simulink model "MX_Gateway"  (Simulink 9.0)
settings: Solver=FixedStepDiscrete  StartTime=0.0  StopTime=100  FixedStep=0.01  RelTol=1e-3
values from neighbouring files (symbols this model uses):
  ACTIVE = Bobcat.Parameter; CreateDefine( ACTIVE                         ,1                          ,'Define' ,'defines.h' )    [defines.m]
  ACTIVE_MODE_ONE = Bobcat.Parameter; CreateDefine( ACTIVE_MODE_ONE                ,19                         ,'Define' ,'defines.h' )    [defines.m]
  AUTHENTICATED = Bobcat.Parameter; CreateDefine( AUTHENTICATED                  ,1                          ,'Define' ,'defines.h' )    [defines.m]
  AUX_EMEA_CONTROLS = Bobcat.Parameter; CreateDefine( AUX_EMEA_CONTROLS  ,1   ,'Define' ,'defines.h' )    [enums.m]
  AUX_FLOW_HIGH = Bobcat.Parameter; CreateDefine( AUX_FLOW_HIGH           ,4  ,'Define' ,'defines.h' )    [enums.m]
  AUX_FLOW_INACTIVE = Bobcat.Parameter; CreateDefine( AUX_FLOW_INACTIVE       ,0  ,'Define' ,'defines.h' )    [enums.m]
  AUX_FLOW_LOW = Bobcat.Parameter; CreateDefine( AUX_FLOW_LOW            ,1  ,'Define' ,'defines.h' )    [enums.m]
  AUX_FLOW_MEDIUM = Bobcat.Parameter; CreateDefine( AUX_FLOW_MEDIUM         ,2  ,'Define' ,'defines.h' )    [enums.m]
  AUX_FLOW_STANDARD = Bobcat.Parameter; CreateDefine( AUX_FLOW_STANDARD       ,3  ,'Define' ,'defines.h' )    [enums.m]
  AUX_NON_FUNCTIONAL = Bobcat.Parameter; CreateDefine( AUX_NON_FUNCTIONAL ,255 ,'Define' ,'defines.h' )    [enums.m]
  BASE_ACTIVE = Bobcat.Parameter; CreateDefine( BASE_ACTIVE                    ,253                        ,'Define' ,'defines.h' )    [defines.m]
  BASE_DETENT = Bobcat.Parameter; CreateDefine( BASE_DETENT                    ,1                          ,'Define' ,'defines.h' )    [defines.m]
  BEEP_NONE = Bobcat.Parameter; CreateDefine( BEEP_NONE                      ,255                        ,'Define' ,'defines.h' )    [defines.m]
  CD_AIR_FILTER_DEBOUNCE_NORMAL = Bobcat.Parameter; CreateAliasCD( CD_AIR_FILTER_DEBOUNCE_NORMAL                        ,uint16(500)                ,'ConstantDataPointer' ,'CONSTANT_DATA' ,'AIR_FILTER_DEBOUNCE_NORMAL')    [cdata.m]    (also in hc12_cdata.m; m4_cdata.m)
  CD_AIR_FILTER_DEBOUNCE_NOT_CONNECTED = Bobcat.Parameter; CreateAliasCD( CD_AIR_FILTER_DEBOUNCE_NOT_CONNECTED                 ,uint16(3000)               ,'ConstantDataPointer' ,'CONSTANT_DATA' ,'AIR_FILTER_DEBOUNCE_NOT_CONNECTED')    [cdata.m]    (also in hc12_cdata.m; m4_cdata.m)
  CD_AIR_FILTER_DEBOUNCE_PLUGGED = Bobcat.Parameter; CreateAliasCD( CD_AIR_FILTER_DEBOUNCE_PLUGGED                       ,uint16(3000)               ,'ConstantDataPointer' ,'CONSTANT_DATA' ,'AIR_FILTER_DEBOUNCE_PLUGGED')    [cdata.m]    (also in hc12_cdata.m; m4_cdata.m)
  CD_AIR_FILTER_FUNCTION = Bobcat.Parameter; CreateAliasCD( CD_AIR_FILTER_FUNCTION                               ,uint8(255)                 ,'ConstantDataPointer' ,'CONSTANT_DATA' ,'AIR_FILTER_FUNCTION')    [cdata.m]    (also in hc12_cdata.m; m4_cdata.m)
  CD_AIR_FILTER_PLUGGED_WEIGHT = Bobcat.Parameter; CreateAliasCD( CD_AIR_FILTER_PLUGGED_WEIGHT                         ,uint8(2)                   ,'ConstantDataPointer' ,'CONSTANT_DATA' ,'AIR_FILTER_PLUGGED_WEIGHT')    [cdata.m]    (also in hc12_cdata.m; m4_cdata.m)
  CD_AIR_FILTER_UNPLUGGED_WEIGHT = Bobcat.Parameter; CreateAliasCD( CD_AIR_FILTER_UNPLUGGED_WEIGHT                       ,uint8(1)                   ,'ConstantDataPointer' ,'CONSTANT_DATA' ,'AIR_FILTER_UNPLUGGED_WEIGHT')    [cdata.m]    (also in hc12_cdata.m; m4_cdata.m)
  CD_AUTOIDLE_ARMBAR_DELAY = Bobcat.Parameter; CreateAliasCD( CD_AUTOIDLE_ARMBAR_DELAY                             ,uint8(2)                   ,'ConstantDataPointer' ,'CONSTANT_DATA' ,'AUTOIDLE_ARMBAR_DELAY')    [cdata.m]    (also in hc12_cdata.m; m4_cdata.m)
  CD_AUTOIDLE_FUNCTION = Bobcat.Parameter; CreateAliasCD( CD_AUTOIDLE_FUNCTION                                 ,uint8(255)                 ,'ConstantDataPointer' ,'CONSTANT_DATA' ,'AUTOIDLE_FUNCTION')    [cdata.m]    (also in hc12_cdata.m; m4_cdata.m)
  CD_AUTOIDLE_IDLE_DELAY = Bobcat.Parameter; CreateAliasCD( CD_AUTOIDLE_IDLE_DELAY                               ,uint8(40)                  ,'ConstantDataPointer' ,'CONSTANT_DATA' ,'AUTOIDLE_IDLE_DELAY')    [cdata.m]    (also in hc12_cdata.m; m4_cdata.m)
  CD_AUTOIDLE_LOW_IDLE_START = Bobcat.Parameter; CreateAliasCD( CD_AUTOIDLE_LOW_IDLE_START                           ,uint8(255)                 ,'ConstantDataPointer' ,'CONSTANT_DATA' ,'AUTOIDLE_LOW_IDLE_START')    [cdata.m]    (also in hc12_cdata.m; m4_cdata.m)
  CD_AUTOIDLE_PRESSURE_OFF = Bobcat.Parameter; CreateAliasCD( CD_AUTOIDLE_PRESSURE_OFF                             ,uint16(300)                ,'ConstantDataPointer' ,'CONSTANT_DATA' ,'AUTOIDLE_PRESSURE_OFF')    [cdata.m]    (also in hc12_cdata.m; m4_cdata.m)
  CD_AUTOIDLE_PRESSURE_ON = Bobcat.Parameter; CreateAliasCD( CD_AUTOIDLE_PRESSURE_ON                              ,uint16(270)                ,'ConstantDataPointer' ,'CONSTANT_DATA' ,'AUTOIDLE_PRESSURE_ON')    [cdata.m]    (also in hc12_cdata.m; m4_cdata.m)
  CD_AUTOSHIFT_FUNCTION = Bobcat.Parameter; CreateAliasCD( CD_AUTOSHIFT_FUNCTION                                ,uint8(255)                 ,'ConstantDataPointer' ,'CONSTANT_DATA' ,'AUTOSHIFT_FUNCTION')    [cdata.m]    (also in hc12_cdata.m; m4_cdata.m)
  CD_AUTOSHIFT_PHIGH1 = Bobcat.Parameter; CreateAliasCD( CD_AUTOSHIFT_PHIGH1                                  ,uint16(205)                ,'ConstantDataPointer' ,'CONSTANT_DATA' ,'AUTOSHIFT_PHIGH1')    [cdata.m]    (also in hc12_cdata.m; m4_cdata.m)
  CD_AUTOSHIFT_PHIGH2 = Bobcat.Parameter; CreateAliasCD( CD_AUTOSHIFT_PHIGH2                                  ,uint16(193)                ,'ConstantDataPointer' ,'CONSTANT_DATA' ,'AUTOSHIFT_PHIGH2')    [cdata.m]    (also in hc12_cdata.m; m4_cdata.m)
  CD_AUTOSHIFT_PHIGH_TEMP1 = Bobcat.Parameter; CreateAliasCD( CD_AUTOSHIFT_PHIGH_TEMP1                             ,int16(70)                  ,'ConstantDataPointer' ,'CONSTANT_DATA' ,'AUTOSHIFT_PHIGH_TEMP1')    [cdata.m]    (also in hc12_cdata.m; m4_cdata.m)
  CD_AUTOSHIFT_PHIGH_TEMP2 = Bobcat.Parameter; CreateAliasCD( CD_AUTOSHIFT_PHIGH_TEMP2                             ,int16(160)                 ,'ConstantDataPointer' ,'CONSTANT_DATA' ,'AUTOSHIFT_PHIGH_TEMP2')    [cdata.m]    (also in hc12_cdata.m; m4_cdata.m)
  CD_AUTOSHIFT_PLOW1 = Bobcat.Parameter; CreateAliasCD( CD_AUTOSHIFT_PLOW1                                   ,uint16(130)                ,'ConstantDataPointer' ,'CONSTANT_DATA' ,'AUTOSHIFT_PLOW1')    [cdata.m]    (also in hc12_cdata.m; m4_cdata.m)
  CD_AUTOSHIFT_PLOW2 = Bobcat.Parameter; CreateAliasCD( CD_AUTOSHIFT_PLOW2                                   ,uint16(100)                ,'ConstantDataPointer' ,'CONSTANT_DATA' ,'AUTOSHIFT_PLOW2')    [cdata.m]    (also in hc12_cdata.m; m4_cdata.m)
  CD_AUTOSHIFT_PLOW_TEMP1 = Bobcat.Parameter; CreateAliasCD( CD_AUTOSHIFT_PLOW_TEMP1                              ,int16(20)                  ,'ConstantDataPointer' ,'CONSTANT_DATA' ,'AUTOSHIFT_PLOW_TEMP1')    [cdata.m]    (also in hc12_cdata.m; m4_cdata.m)
  CD_AUTOSHIFT_PLOW_TEMP2 = Bobcat.Parameter; CreateAliasCD( CD_AUTOSHIFT_PLOW_TEMP2                              ,int16(80)                  ,'ConstantDataPointer' ,'CONSTANT_DATA' ,'AUTOSHIFT_PLOW_TEMP2')    [cdata.m]    (also in hc12_cdata.m; m4_cdata.m)
  CD_AUTOSHIFT_TRAVEL_DELAY = Bobcat.Parameter; CreateAliasCD( CD_AUTOSHIFT_TRAVEL_DELAY                            ,uint8(32)                  ,'ConstantDataPointer' ,'CONSTANT_DATA' ,'AUTOSHIFT_TRAVEL_DELAY')    [cdata.m]    (also in hc12_cdata.m; m4_cdata.m)
  CD_AUX2_CRACKPOINT_CURRENT = Bobcat.Parameter; CreateAliasCD( CD_AUX2_CRACKPOINT_CURRENT                           ,fi(0,0,16,12,'hex','0c28') ,'ConstantDataPointer' ,'CONSTANT_DATA' ,'AUX2_CRACKPOINT_CURRENT')    [cdata.m]    (also in hc12_cdata.m; m4_cdata.m)
  CD_AUX2_FLOW_LOW_FUNCTION = Bobcat.Parameter; CreateAliasCD( CD_AUX2_FLOW_LOW_FUNCTION                            ,uint8(255)                 ,'ConstantDataPointer' ,'CONSTANT_DATA' ,'AUX2_FLOW_LOW_FUNCTION')    [cdata.m]    (also in hc12_cdata.m; m4_cdata.m)
  CD_AUX2_FLOW_MED_FUNCTION = Bobcat.Parameter; CreateAliasCD( CD_AUX2_FLOW_MED_FUNCTION                            ,uint8(255)                 ,'ConstantDataPointer' ,'CONSTANT_DATA' ,'AUX2_FLOW_MED_FUNCTION')    [cdata.m]    (also in hc12_cdata.m; m4_cdata.m)
  CD_AUX2_HI_FLOW_PERCENT = Bobcat.Parameter; CreateAliasCD( CD_AUX2_HI_FLOW_PERCENT                              ,fi(0,0,16,16,'hex','ffff') ,'ConstantDataPointer' ,'CONSTANT_DATA' ,'AUX2_HI_FLOW_PERCENT')    [cdata.m]    (also in hc12_cdata.m; m4_cdata.m)
  CD_AUX2_LOW_FLOW_PERCENT = Bobcat.Parameter; CreateAliasCD( CD_AUX2_LOW_FLOW_PERCENT                             ,fi(0,0,16,16,'hex','7333') ,'ConstantDataPointer' ,'CONSTANT_DATA' ,'AUX2_LOW_FLOW_PERCENT')    [cdata.m]    (also in hc12_cdata.m; m4_cdata.m)
  CD_AUX2_MAX_FLOW_CURRENT = Bobcat.Parameter; CreateAliasCD( CD_AUX2_MAX_FLOW_CURRENT                             ,fi(0,0,16,12,'hex','14cc') ,'ConstantDataPointer' ,'CONSTANT_DATA' ,'AUX2_MAX_FLOW_CURRENT')    [cdata.m]    (also in hc12_cdata.m; m4_cdata.m)
  CD_AUX2_MED_FLOW_PERCENT = Bobcat.Parameter; CreateAliasCD( CD_AUX2_MED_FLOW_PERCENT                             ,fi(0,0,16,16,'hex','9999') ,'ConstantDataPointer' ,'CONSTANT_DATA' ,'AUX2_MED_FLOW_PERCENT')    [cdata.m]    (also in hc12_cdata.m; m4_cdata.m)
  CD_AUX_CRACKPOINT_CURRENT = Bobcat.Parameter; CreateAliasCD( CD_AUX_CRACKPOINT_CURRENT                            ,fi(0,0,16,12,'hex','0000') ,'ConstantDataPointer' ,'CONSTANT_DATA' ,'AUX_CRACKPOINT_CURRENT')    [cdata.m]    (also in hc12_cdata.m; m4_cdata.m)
  CD_AUX_DETENT_FLASH_TIME = Bobcat.Parameter; CreateAliasCD( CD_AUX_DETENT_FLASH_TIME                             ,uint16(72)                 ,'ConstantDataPointer' ,'CONSTANT_DATA' ,'AUX_DETENT_FLASH_TIME')    [cdata.m]    (also in hc12_cdata.m; m4_cdata.m)
  CD_AUX_DETENT_HOLD_TIME = Bobcat.Parameter; CreateAliasCD( CD_AUX_DETENT_HOLD_TIME                              ,uint16(100)                ,'ConstantDataPointer' ,'CONSTANT_DATA' ,'AUX_DETENT_HOLD_TIME')    [cdata.m]    (also in hc12_cdata.m; m4_cdata.m)
  CD_AUX_FULL_ON_AUX1 = Bobcat.Parameter; CreateAliasCD( CD_AUX_FULL_ON_AUX1                                  ,fi(0,0,16,12,'hex','10cd') ,'ConstantDataPointer' ,'CONSTANT_DATA' ,'AUX_FULL_ON_AUX1')    [cdata.m]    (also in hc12_cdata.m; m4_cdata.m)
  CD_AUX_FULL_ON_AUX2 = Bobcat.Parameter; CreateAliasCD( CD_AUX_FULL_ON_AUX2                                  ,fi(0,0,16,12,'hex','139d') ,'ConstantDataPointer' ,'CONSTANT_DATA' ,'AUX_FULL_ON_AUX2')    [cdata.m]    (also in hc12_cdata.m; m4_cdata.m)
  CD_AUX_FULL_ON_AUX3 = Bobcat.Parameter; CreateAliasCD( CD_AUX_FULL_ON_AUX3                                  ,fi(0,0,16,12,'hex','17d7') ,'ConstantDataPointer' ,'CONSTANT_DATA' ,'AUX_FULL_ON_AUX3')    [cdata.m]    (also in hc12_cdata.m; m4_cdata.m)
  CD_AUX_FUNCTION = Bobcat.Parameter; CreateAliasCD( CD_AUX_FUNCTION                                      ,uint8(0)                   ,'ConstantDataPointer' ,'CONSTANT_DATA' ,'AUX_FUNCTION')    [cdata.m]    (also in hc12_cdata.m; m4_cdata.m)
  CD_AUX_RATE_LIMIT_CRACKPOINT = Bobcat.Parameter; CreateAliasCD( CD_AUX_RATE_LIMIT_CRACKPOINT                         ,fi(0,0,16,12,'hex','0800') ,'ConstantDataPointer' ,'CONSTANT_DATA' ,'AUX_RATE_LIMIT_CRACKPOINT')    [cdata.m]    (also in hc12_cdata.m; m4_cdata.m)
  CD_AUX_RATE_LIMIT_CRACKPOINT_RATE = Bobcat.Parameter; CreateAliasCD( CD_AUX_RATE_LIMIT_CRACKPOINT_RATE                    ,fi(0,1,32,12,'int',102400) ,'ConstantDataPointer' ,'CONSTANT_DATA' ,'AUX_RATE_LIMIT_CRACKPOINT_RATE')    [cdata.m]    (also in hc12_cdata.m; m4_cdata.m)
  CD_AUX_RATE_LIMIT_FUNCTION = Bobcat.Parameter; CreateAliasCD( CD_AUX_RATE_LIMIT_FUNCTION                           ,uint8(255)                 ,'ConstantDataPointer' ,'CONSTANT_DATA' ,'AUX_RATE_LIMIT_FUNCTION')    [cdata.m]    (also in hc12_cdata.m; m4_cdata.m)
  CD_AUX_RATE_LIMIT_MAX_ENG_SPD = Bobcat.Parameter; CreateAliasCD( CD_AUX_RATE_LIMIT_MAX_ENG_SPD                        ,uint16(1600)               ,'ConstantDataPointer' ,'CONSTANT_DATA' ,'AUX_RATE_LIMIT_MAX_ENG_SPD')    [cdata.m]    (also in hc12_cdata.m; m4_cdata.m)
  CD_AUX_RATE_LIMIT_MIN_ENG_SPD = Bobcat.Parameter; CreateAliasCD( CD_AUX_RATE_LIMIT_MIN_ENG_SPD                        ,uint16(100)                ,'ConstantDataPointer' ,'CONSTANT_DATA' ,'AUX_RATE_LIMIT_MIN_ENG_SPD')    [cdata.m]    (also in hc12_cdata.m; m4_cdata.m)
  CD_AUX_RATE_LIMIT_NORMAL_RATE = Bobcat.Parameter; CreateAliasCD( CD_AUX_RATE_LIMIT_NORMAL_RATE                        ,fi(0,1,32,12,'int',2048)   ,'ConstantDataPointer' ,'CONSTANT_DATA' ,'AUX_RATE_LIMIT_NORMAL_RATE')    [cdata.m]    (also in hc12_cdata.m; m4_cdata.m)
  CD_AUXPOT_CAL_DEADBAND_HIGH = Bobcat.Parameter; CreateAliasCD( CD_AUXPOT_CAL_DEADBAND_HIGH                          ,uint16(574)                ,'ConstantDataPointer' ,'CONSTANT_DATA' ,'AUXPOT_CAL_DEADBAND_HIGH')    [cdata.m]    (also in hc12_cdata.m; m4_cdata.m)
  CD_AUXPOT_CAL_DEADBAND_LOW = Bobcat.Parameter; CreateAliasCD( CD_AUXPOT_CAL_DEADBAND_LOW                           ,uint16(424)                ,'ConstantDataPointer' ,'CONSTANT_DATA' ,'AUXPOT_CAL_DEADBAND_LOW')    [cdata.m]    (also in hc12_cdata.m; m4_cdata.m)
  CD_AUXPOT_CENTER_RATIO = Bobcat.Parameter; CreateAliasCD( CD_AUXPOT_CENTER_RATIO                               ,uint16(500)                ,'ConstantDataPointer' ,'CONSTANT_DATA' ,'AUXPOT_CENTER_RATIO')    [cdata.m]    (also in hc12_cdata.m; m4_cdata.m)
  CD_AUXPOT_DEADBAND = Bobcat.Parameter; CreateAliasCD( CD_AUXPOT_DEADBAND                                   ,uint8(38)                  ,'ConstantDataPointer' ,'CONSTANT_DATA' ,'AUXPOT_DEADBAND')    [cdata.m]    (also in hc12_cdata.m; m4_cdata.m)
  CD_AUXPOT_FUNCTION = Bobcat.Parameter; CreateAliasCD( CD_AUXPOT_FUNCTION                                   ,uint8(0)                   ,'ConstantDataPointer' ,'CONSTANT_DATA' ,'AUXPOT_FUNCTION')    [cdata.m]    (also in hc12_cdata.m; m4_cdata.m)
  CD_AUXPOT_RATIO_GAIN = Bobcat.Parameter; CreateAliasCD( CD_AUXPOT_RATIO_GAIN                                 ,uint16(1000)               ,'ConstantDataPointer' ,'CONSTANT_DATA' ,'AUXPOT_RATIO_GAIN')    [cdata.m]    (also in hc12_cdata.m; m4_cdata.m)
  CD_CAN_THROTTLE_FUNCTION = Bobcat.Parameter; CreateAliasCD( CD_CAN_THROTTLE_FUNCTION                             ,uint8(255)                 ,'ConstantDataPointer' ,'CONSTANT_DATA' ,'CAN_THROTTLE_FUNCTION')    [cdata.m]    (also in hc12_cdata.m; m4_cdata.m)
  CD_CAN_THROTTLE_REVERSE = Bobcat.Parameter; CreateAliasCD( CD_CAN_THROTTLE_REVERSE                              ,uint8(255)                 ,'ConstantDataPointer' ,'CONSTANT_DATA' ,'CAN_THROTTLE_REVERSE')    [cdata.m]    (also in hc12_cdata.m; m4_cdata.m)
  CD_CONSOLE_DEBOUNCE_ABSENT = Bobcat.Parameter; CreateAliasCD( CD_CONSOLE_DEBOUNCE_ABSENT                           ,uint16(12)                 ,'ConstantDataPointer' ,'CONSTANT_DATA' ,'CONSOLE_DEBOUNCE_ABSENT')    [cdata.m]    (also in hc12_cdata.m; m4_cdata.m)
  CD_CONSOLE_DEBOUNCE_OORH = Bobcat.Parameter; CreateAliasCD( CD_CONSOLE_DEBOUNCE_OORH                             ,uint16(12)                 ,'ConstantDataPointer' ,'CONSTANT_DATA' ,'CONSOLE_DEBOUNCE_OORH')    [cdata.m]    (also in hc12_cdata.m; m4_cdata.m)
  CD_CONSOLE_DEBOUNCE_OORL = Bobcat.Parameter; CreateAliasCD( CD_CONSOLE_DEBOUNCE_OORL                             ,uint16(12)                 ,'ConstantDataPointer' ,'CONSTANT_DATA' ,'CONSOLE_DEBOUNCE_OORL')    [cdata.m]    (also in hc12_cdata.m; m4_cdata.m)
  CD_CONSOLE_DEBOUNCE_PRESENT = Bobcat.Parameter; CreateAliasCD( CD_CONSOLE_DEBOUNCE_PRESENT                          ,uint16(12)                 ,'ConstantDataPointer' ,'CONSTANT_DATA' ,'CONSOLE_DEBOUNCE_PRESENT')    [cdata.m]    (also in hc12_cdata.m; m4_cdata.m)
  CD_CONSOLE_FUNCTION = Bobcat.Parameter; CreateAliasCD( CD_CONSOLE_FUNCTION                                  ,uint8(0)                   ,'ConstantDataPointer' ,'CONSTANT_DATA' ,'CONSOLE_FUNCTION')    [cdata.m]    (also in hc12_cdata.m; m4_cdata.m)
  CD_CONSOLE_OORH = Bobcat.Parameter; CreateAliasCD( CD_CONSOLE_OORH                                      ,uint16(740)                ,'ConstantDataPointer' ,'CONSTANT_DATA' ,'CONSOLE_OORH')    [cdata.m]    (also in hc12_cdata.m; m4_cdata.m)
  CD_CONSOLE_OORL = Bobcat.Parameter; CreateAliasCD( CD_CONSOLE_OORL                                      ,uint16(10)                 ,'ConstantDataPointer' ,'CONSTANT_DATA' ,'CONSOLE_OORL')    [cdata.m]    (also in hc12_cdata.m; m4_cdata.m)
  CD_CONSOLE_PRESENT_HIGH = Bobcat.Parameter; CreateAliasCD( CD_CONSOLE_PRESENT_HIGH                              ,uint16(515)                ,'ConstantDataPointer' ,'CONSTANT_DATA' ,'CONSOLE_PRESENT_HIGH')    [cdata.m]    (also in hc12_cdata.m; m4_cdata.m)
  CD_CONSOLE_PRESENT_LOW = Bobcat.Parameter; CreateAliasCD( CD_CONSOLE_PRESENT_LOW                               ,uint16(375)                ,'ConstantDataPointer' ,'CONSTANT_DATA' ,'CONSOLE_PRESENT_LOW')    [cdata.m]    (also in hc12_cdata.m; m4_cdata.m)
  CD_DELUXE_GEN5_5_FUNCTION = Bobcat.Parameter; CreateAliasCD( CD_DELUXE_GEN5_5_FUNCTION                            ,uint8(255)                 ,'ConstantDataPointer' ,'CONSTANT_DATA' ,'DELUXE_GEN5_5_FUNCTION')    [cdata.m]    (also in hc12_cdata.m; m4_cdata.m)
  CD_DELUXE_GEN5_FUNCTION = Bobcat.Parameter; CreateAliasCD( CD_DELUXE_GEN5_FUNCTION                              ,uint8(255)                 ,'ConstantDataPointer' ,'CONSTANT_DATA' ,'DELUXE_GEN5_FUNCTION')    [cdata.m]    (also in hc12_cdata.m; m4_cdata.m)
  CD_DERATE_ENGINE_SPEED_DEBOUNCE_HIGH = Bobcat.Parameter; CreateAliasCD( CD_DERATE_ENGINE_SPEED_DEBOUNCE_HIGH                 ,uint16(200)                ,'ConstantDataPointer' ,'CONSTANT_DATA' ,'DERATE_ENGINE_SPEED_DEBOUNCE_HIGH')    [cdata.m]    (also in hc12_cdata.m; m4_cdata.m)
  CD_DERATE_ENGINE_SPEED_DEBOUNCE_SHUTDOWN = Bobcat.Parameter; CreateAliasCD( CD_DERATE_ENGINE_SPEED_DEBOUNCE_SHUTDOWN             ,uint16(1000)               ,'ConstantDataPointer' ,'CONSTANT_DATA' ,'DERATE_ENGINE_SPEED_DEBOUNCE_SHUTDOWN')    [cdata.m]    (also in hc12_cdata.m; m4_cdata.m)
  CD_DERATE_ENGINE_SPEED_HIGH_OFFSET = Bobcat.Parameter; CreateAliasCD( CD_DERATE_ENGINE_SPEED_HIGH_OFFSET                   ,uint16(200)                ,'ConstantDataPointer' ,'CONSTANT_DATA' ,'DERATE_ENGINE_SPEED_HIGH_OFFSET')    [cdata.m]    (also in hc12_cdata.m; m4_cdata.m)
  CD_DERATE_METHOD = Bobcat.Parameter; CreateAliasCD( CD_DERATE_METHOD                                     ,uint8(0)                   ,'ConstantDataPointer' ,'CONSTANT_DATA' ,'DERATE_METHOD')    [cdata.m]    (also in hc12_cdata.m; m4_cdata.m)
  CD_DUMP_VALVE_FUNCTION = Bobcat.Parameter; CreateAliasCD( CD_DUMP_VALVE_FUNCTION                               ,uint8(255)                 ,'ConstantDataPointer' ,'CONSTANT_DATA' ,'DUMP_VALVE_FUNCTION')    [cdata.m]    (also in hc12_cdata.m; m4_cdata.m)
  CD_DUMP_VALVE_ON_CURRENT = Bobcat.Parameter; CreateAliasCD( CD_DUMP_VALVE_ON_CURRENT                             ,fi(0,0,16,12,'hex','ffff') ,'ConstantDataPointer' ,'CONSTANT_DATA' ,'DUMP_VALVE_ON_CURRENT')    [cdata.m]    (also in hc12_cdata.m; m4_cdata.m)
  CD_ECO_MODE_THROTTLE_REDUCTION = Bobcat.Parameter; CreateAliasCD( CD_ECO_MODE_THROTTLE_REDUCTION                       ,uint16(850)                ,'ConstantDataPointer' ,'CONSTANT_DATA' ,'ECO_MODE_THROTTLE_REDUCTION')    [cdata.m]    (also in hc12_cdata.m; m4_cdata.m)
  CD_ECT_FILTER_GAIN = Bobcat.Parameter; CreateAliasCD( CD_ECT_FILTER_GAIN                                   ,uint8(6)                   ,'ConstantDataPointer' ,'CONSTANT_DATA' ,'ECT_FILTER_GAIN')    [cdata.m]    (also in hc12_cdata.m; m4_cdata.m)
  CD_ECU_FUNCTION = Bobcat.Parameter; CreateAliasCD( CD_ECU_FUNCTION                                      ,uint8(255)                 ,'ConstantDataPointer' ,'CONSTANT_DATA' ,'ECU_FUNCTION')    [cdata.m]    (also in hc12_cdata.m; m4_cdata.m)
  CD_ECU_HOLD_DEBOUNCE_ERROR_ON = Bobcat.Parameter; CreateAliasCD( CD_ECU_HOLD_DEBOUNCE_ERROR_ON                        ,uint16(20)                 ,'ConstantDataPointer' ,'CONSTANT_DATA' ,'ECU_HOLD_DEBOUNCE_ERROR_ON')    [cdata.m]    (also in hc12_cdata.m; m4_cdata.m)
  CD_EMEA_CONTROLS_HOLD_TIME = Bobcat.Parameter; CreateAliasCD( CD_EMEA_CONTROLS_HOLD_TIME                           ,uint16(30)                 ,'ConstantDataPointer' ,'CONSTANT_DATA' ,'EMEA_CONTROLS_HOLD_TIME')    [cdata.m]    (also in hc12_cdata.m; m4_cdata.m)
  CD_EMEA_CONTROLS_OPTION_A = Bobcat.Parameter; CreateAliasCD( CD_EMEA_CONTROLS_OPTION_A                            ,uint8(255)                 ,'ConstantDataPointer' ,'CONSTANT_DATA' ,'EMEA_CONTROLS_OPTION_A')    [cdata.m]    (also in hc12_cdata.m; m4_cdata.m)
  CD_EMEA_CONTROLS_OPTION_C = Bobcat.Parameter; CreateAliasCD( CD_EMEA_CONTROLS_OPTION_C                            ,uint8(255)                 ,'ConstantDataPointer' ,'CONSTANT_DATA' ,'EMEA_CONTROLS_OPTION_C')    [cdata.m]    (also in hc12_cdata.m; m4_cdata.m)
  CD_EMEA_CONTROLS_REVERSE = Bobcat.Parameter; CreateAliasCD( CD_EMEA_CONTROLS_REVERSE                             ,uint8(255)                 ,'ConstantDataPointer' ,'CONSTANT_DATA' ,'EMEA_CONTROLS_REVERSE')    [cdata.m]    (also in hc12_cdata.m; m4_cdata.m)
  CD_EMEA_ELECTRIC_VERSION = Bobcat.Parameter; CreateAliasCD( CD_EMEA_ELECTRIC_VERSION                             ,uint8(0)                   ,'ConstantDataPointer' ,'CONSTANT_DATA' ,'EMEA_ELECTRIC_VERSION')    [cdata.m]    (also in hc12_cdata.m; m4_cdata.m)
  CD_EMEA_POT_CAL_DEADBAND_HIGH = Bobcat.Parameter; CreateAliasCD( CD_EMEA_POT_CAL_DEADBAND_HIGH                        ,uint16(574)                ,'ConstantDataPointer' ,'CONSTANT_DATA' ,'EMEA_POT_CAL_DEADBAND_HIGH')    [cdata.m]    (also in hc12_cdata.m; m4_cdata.m)
  CD_EMEA_POT_CAL_DEADBAND_LOW = Bobcat.Parameter; CreateAliasCD( CD_EMEA_POT_CAL_DEADBAND_LOW                         ,uint16(424)                ,'ConstantDataPointer' ,'CONSTANT_DATA' ,'EMEA_POT_CAL_DEADBAND_LOW')    [cdata.m]    (also in hc12_cdata.m; m4_cdata.m)
  CD_ENGINE_RPM_FACTOR = Bobcat.Parameter; CreateAliasCD( CD_ENGINE_RPM_FACTOR                                 ,uint8(35)                  ,'ConstantDataPointer' ,'CONSTANT_DATA' ,'ENGINE_RPM_FACTOR')    [cdata.m]    (also in hc12_cdata.m; m4_cdata.m)
  CD_ENGINE_SPEED_FILTER_FAST_VALUE = Bobcat.Parameter; CreateAliasCD( CD_ENGINE_SPEED_FILTER_FAST_VALUE                    ,uint8(1)                   ,'ConstantDataPointer' ,'CONSTANT_DATA' ,'ENGINE_SPEED_FILTER_FAST_VALUE')    [cdata.m]    (also in hc12_cdata.m; m4_cdata.m)
  CD_ENGINE_SPEED_FILTER_SLOW_VALUE = Bobcat.Parameter; CreateAliasCD( CD_ENGINE_SPEED_FILTER_SLOW_VALUE                    ,uint8(4)                   ,'ConstantDataPointer' ,'CONSTANT_DATA' ,'ENGINE_SPEED_FILTER_SLOW_VALUE')    [cdata.m]    (also in hc12_cdata.m; m4_cdata.m)
  CD_ENGINE_SPEED_RUNNING = Bobcat.Parameter; CreateAliasCD( CD_ENGINE_SPEED_RUNNING                              ,uint16(800)                ,'ConstantDataPointer' ,'CONSTANT_DATA' ,'ENGINE_SPEED_RUNNING')    [cdata.m]    (also in hc12_cdata.m; m4_cdata.m)
  CD_ENGINE_START_DELAY_DEBOUNCE_RPM_NO_SIGNAL = Bobcat.Parameter; CreateAliasCD( CD_ENGINE_START_DELAY_DEBOUNCE_RPM_NO_SIGNAL         ,uint16(0)                  ,'ConstantDataPointer' ,'CONSTANT_DATA' ,'ENGINE_START_DELAY_DEBOUNCE_RPM_NO_SIGNAL')    [cdata.m]    (also in hc12_cdata.m; m4_cdata.m)
  CD_ENGINE_START_DELAY_OFF_TIME = Bobcat.Parameter; CreateAliasCD( CD_ENGINE_START_DELAY_OFF_TIME                       ,uint16(0)                  ,'ConstantDataPointer' ,'CONSTANT_DATA' ,'ENGINE_START_DELAY_OFF_TIME')    [cdata.m]    (also in hc12_cdata.m; m4_cdata.m)
  CD_ENGINE_START_DELAY_RPM_NO_SIGNAL = Bobcat.Parameter; CreateAliasCD( CD_ENGINE_START_DELAY_RPM_NO_SIGNAL                  ,uint16(0)                  ,'ConstantDataPointer' ,'CONSTANT_DATA' ,'ENGINE_START_DELAY_RPM_NO_SIGNAL')    [cdata.m]    (also in hc12_cdata.m; m4_cdata.m)
  CD_EOP_DEBOUNCE_EXTREMELY_LOW = Bobcat.Parameter; CreateAliasCD( CD_EOP_DEBOUNCE_EXTREMELY_LOW                        ,uint16(50)                 ,'ConstantDataPointer' ,'CONSTANT_DATA' ,'EOP_DEBOUNCE_EXTREMELY_LOW')    [cdata.m]    (also in hc12_cdata.m; m4_cdata.m)
  CD_EOP_DEBOUNCE_NORMAL = Bobcat.Parameter; CreateAliasCD( CD_EOP_DEBOUNCE_NORMAL                               ,uint16(50)                 ,'ConstantDataPointer' ,'CONSTANT_DATA' ,'EOP_DEBOUNCE_NORMAL')    [cdata.m]    (also in hc12_cdata.m; m4_cdata.m)
  CD_EOP_DEBOUNCE_SHUTDOWN = Bobcat.Parameter; CreateAliasCD( CD_EOP_DEBOUNCE_SHUTDOWN                             ,uint16(100)                ,'ConstantDataPointer' ,'CONSTANT_DATA' ,'EOP_DEBOUNCE_SHUTDOWN')    [cdata.m]    (also in hc12_cdata.m; m4_cdata.m)
  CD_EOP_FILTER_VALUE = Bobcat.Parameter; CreateAliasCD( CD_EOP_FILTER_VALUE                                  ,uint8(3)                   ,'ConstantDataPointer' ,'CONSTANT_DATA' ,'EOP_FILTER_VALUE')    [cdata.m]    (also in hc12_cdata.m; m4_cdata.m)
  CD_EOP_FUNCTION = Bobcat.Parameter; CreateAliasCD( CD_EOP_FUNCTION                                      ,uint8(1)                   ,'ConstantDataPointer' ,'CONSTANT_DATA' ,'EOP_FUNCTION')    [cdata.m]    (also in hc12_cdata.m; m4_cdata.m)
  CD_EOP_SWITCH_GAUGE_VALUE = Bobcat.Parameter; CreateAliasCD( CD_EOP_SWITCH_GAUGE_VALUE                            ,uint16(125)                ,'ConstantDataPointer' ,'CONSTANT_DATA' ,'EOP_SWITCH_GAUGE_VALUE')    [cdata.m]    (also in hc12_cdata.m; m4_cdata.m)
  CD_EOP_SWITCH_LOW = Bobcat.Parameter; CreateAliasCD( CD_EOP_SWITCH_LOW                                    ,uint16(50)                 ,'ConstantDataPointer' ,'CONSTANT_DATA' ,'EOP_SWITCH_LOW')    [cdata.m]    (also in hc12_cdata.m; m4_cdata.m)
  CD_EOP_SWITCH_PRESSURE_VALUE = Bobcat.Parameter; CreateAliasCD( CD_EOP_SWITCH_PRESSURE_VALUE                         ,uint16(40)                 ,'ConstantDataPointer' ,'CONSTANT_DATA' ,'EOP_SWITCH_PRESSURE_VALUE')    [cdata.m]    (also in hc12_cdata.m; m4_cdata.m)
  CD_EOT_DEBOUNCE_EXTREMELY_LOW = Bobcat.Parameter; CreateAliasCD( CD_EOT_DEBOUNCE_EXTREMELY_LOW                        ,uint16(2)                  ,'ConstantDataPointer' ,'CONSTANT_DATA' ,'EOT_DEBOUNCE_EXTREMELY_LOW')    [cdata.m]    (also in hc12_cdata.m; m4_cdata.m)
  CD_EOT_DEBOUNCE_LOW = Bobcat.Parameter; CreateAliasCD( CD_EOT_DEBOUNCE_LOW                                  ,uint16(2)                  ,'ConstantDataPointer' ,'CONSTANT_DATA' ,'EOT_DEBOUNCE_LOW')    [cdata.m]    (also in hc12_cdata.m; m4_cdata.m)
  CD_EOT_DEBOUNCE_NORMAL = Bobcat.Parameter; CreateAliasCD( CD_EOT_DEBOUNCE_NORMAL                               ,uint16(2)                  ,'ConstantDataPointer' ,'CONSTANT_DATA' ,'EOT_DEBOUNCE_NORMAL')    [cdata.m]    (also in hc12_cdata.m; m4_cdata.m)
  CD_EOT_EXTREMELY_LOW = Bobcat.Parameter; CreateAliasCD( CD_EOT_EXTREMELY_LOW                                 ,uint16(81)                 ,'ConstantDataPointer' ,'CONSTANT_DATA' ,'EOT_EXTREMELY_LOW')    [cdata.m]    (also in hc12_cdata.m; m4_cdata.m)
  CD_EOT_FUNCTION = Bobcat.Parameter; CreateAliasCD( CD_EOT_FUNCTION                                      ,uint8(255)                 ,'ConstantDataPointer' ,'CONSTANT_DATA' ,'EOT_FUNCTION')    [cdata.m]    (also in hc12_cdata.m; m4_cdata.m)
  CD_EOT_LOW = Bobcat.Parameter; CreateAliasCD( CD_EOT_LOW                                           ,uint16(90)                 ,'ConstantDataPointer' ,'CONSTANT_DATA' ,'EOT_LOW')    [cdata.m]    (also in hc12_cdata.m; m4_cdata.m)
  CD_EOT_TEMPERATURE_HYSTERESIS = Bobcat.Parameter; CreateAliasCD( CD_EOT_TEMPERATURE_HYSTERESIS                        ,uint16(2)                  ,'ConstantDataPointer' ,'CONSTANT_DATA' ,'EOT_TEMPERATURE_HYSTERESIS')    [cdata.m]    (also in hc12_cdata.m; m4_cdata.m)
  CD_FAST_TRAVEL_FUNCTION = Bobcat.Parameter; CreateAliasCD( CD_FAST_TRAVEL_FUNCTION                              ,uint8(255)                 ,'ConstantDataPointer' ,'CONSTANT_DATA' ,'FAST_TRAVEL_FUNCTION')    [cdata.m]    (also in hc12_cdata.m; m4_cdata.m)
  CD_FAST_TRAVEL_THROTTLE_DELAY = Bobcat.Parameter; CreateAliasCD( CD_FAST_TRAVEL_THROTTLE_DELAY                        ,uint16(10)                 ,'ConstantDataPointer' ,'CONSTANT_DATA' ,'FAST_TRAVEL_THROTTLE_DELAY')    [cdata.m]    (also in hc12_cdata.m; m4_cdata.m)
  CD_FAST_TRAVEL_THROTTLE_LIMIT = Bobcat.Parameter; CreateAliasCD( CD_FAST_TRAVEL_THROTTLE_LIMIT                        ,uint16(1000)               ,'ConstantDataPointer' ,'CONSTANT_DATA' ,'FAST_TRAVEL_THROTTLE_LIMIT')    [cdata.m]    (also in hc12_cdata.m; m4_cdata.m)
  CD_FLYWHEEL_TEETH = Bobcat.Parameter; CreateAliasCD( CD_FLYWHEEL_TEETH                                    ,uint8(83)                  ,'ConstantDataPointer' ,'CONSTANT_DATA' ,'FLYWHEEL_TEETH')    [cdata.m]    (also in hc12_cdata.m; m4_cdata.m)
  CD_FUEL_LEVEL_FILTER_FAST_VALUE = Bobcat.Parameter; CreateAliasCD( CD_FUEL_LEVEL_FILTER_FAST_VALUE                      ,uint8(5)                   ,'ConstantDataPointer' ,'CONSTANT_DATA' ,'FUEL_LEVEL_FILTER_FAST_VALUE')    [cdata.m]    (also in hc12_cdata.m; m4_cdata.m)
  CD_FUEL_LEVEL_FILTER_SLOW_VALUE = Bobcat.Parameter; CreateAliasCD( CD_FUEL_LEVEL_FILTER_SLOW_VALUE                      ,uint8(10)                  ,'ConstantDataPointer' ,'CONSTANT_DATA' ,'FUEL_LEVEL_FILTER_SLOW_VALUE')    [cdata.m]    (also in hc12_cdata.m; m4_cdata.m)
  CD_FUEL_PRIMARY_FEEDBACK_FUNCTION = Bobcat.Parameter; CreateAliasCD( CD_FUEL_PRIMARY_FEEDBACK_FUNCTION                    ,uint8(0)                   ,'ConstantDataPointer' ,'CONSTANT_DATA' ,'FUEL_PRIMARY_FEEDBACK_FUNCTION')    [cdata.m]    (also in hc12_cdata.m; m4_cdata.m)
  ... (+246 more)
undefined symbols (not defined in the file or in its folder): sigbuilder_block
undefined symbols (not defined in the file): CD_FUEL_RESOLUTION_TIMER
libraries: simulink x186, MX_Library x107
(part 34 of 48)

== stateflow chart MX_Gtwy_Control/Outputs/Two_Speed/Two_Speed/TwoSpeed_Control ==
  state Control [parallel]
    settings: executionOrder=1
    state NonFunctional
      en:
      cmd_TwoSpeed = INACTIVE;
      state_TwoSpeedIcon = INACTIVE;
    state AutoShift_TrackTensioning
      state Inactive
        en:
        cmd_TwoSpeed = INACTIVE;
        state_TwoSpeedIcon = INACTIVE;
      state Active
        state Low_Range
          en:
          state_TwoSpeedIcon = INACTIVE;
          state Normal
            en:
            cmd_TwoSpeed = LOW;
          state Track_Tensioning
            en:
            cmd_TwoSpeed = HIGH;
          (default) -> Control.AutoShift_TrackTensioning.Active.Low_Range.Normal
          Control.AutoShift_TrackTensioning.Active.Low_Range.Normal -> Control.AutoShift_TrackTensioning.Active.Low_Range.Track_Tensioning : [din_TravelPressureSwitch == INACTIVE]
          Control.AutoShift_TrackTensioning.Active.Low_Range.Track_Tensioning -> Control.AutoShift_TrackTensioning.Active.Low_Range.Normal : [din_TravelPressureSwitch == ACTIVE]
        state High_Range_Available
          en:
          state_TwoSpeedIcon = ACTIVE;
          state Travel_Autoshift
            state Low_Delay
              en:
              cmd_TwoSpeed = LOW;
            state High
              en:
              cmd_TwoSpeed = HIGH;
            state Low_Travel
              en:
              cmd_TwoSpeed = LOW;
            (default) -> Low_Delay
            Low_Delay -> Control.AutoShift_TrackTensioning.Active.High_Range_Available.Travel_Autoshift.High : [after(CD_AUTOSHIFT_TRAVEL_DELAY, tick)]
            Control.AutoShift_TrackTensioning.Active.High_Range_Available.Travel_Autoshift.High -> Control.AutoShift_TrackTensioning.Active.High_Range_Available.Travel_Autoshift.Low_Travel : [pressure_LoadSense > P_high...
                | && state_LoadSense == NORMAL]
            Control.AutoShift_TrackTensioning.Active.High_Range_Available.Travel_Autoshift.Low_Travel -> Control.AutoShift_TrackTensioning.Active.High_Range_Available.Travel_Autoshift.High : [pressure_LoadSense < P_low...
                | || state_LoadSense != NORMAL]
          state NoTravel_High
            en:
            cmd_TwoSpeed = HIGH;
          (default) -> NoTravel_High
          NoTravel_High -> Travel_Autoshift : [din_TravelPressureSwitch == ACTIVE]
          Travel_Autoshift -> NoTravel_High : [din_TravelPressureSwitch == INACTIVE]
        (default) -> Low_Range
        Low_Range -> (junction 2666) : [risingedge_TwoSpeed]
        (junction 2668) -> Low_Range [order 2]
        (junction 2669) -> Low_Range : {
            | state_Buzzer = SINGLE_SHORT_BEEP;
            | }
        (junction 2666) -> (junction 2667) [order 1] : [CD_TWOSPEED_BEEP_FUNCTION != NON_FUNCTIONAL]
        (junction 2668) -> (junction 2669) [order 1] : [CD_TWOSPEED_BEEP_FUNCTION != NON_FUNCTIONAL]
        (junction 2666) -> High_Range_Available [order 2]
        (junction 2667) -> High_Range_Available : {
            | state_Buzzer = DOUBLE_SHORT_BEEP;
            | }
        High_Range_Available -> (junction 2668) : [risingedge_TwoSpeed]
      (default) -> Control.AutoShift_TrackTensioning.Inactive
      Control.AutoShift_TrackTensioning.Inactive -> Control.AutoShift_TrackTensioning.Active : [state_Console == ACTIVE...
          | && state_HOT != HIGH...
          | && state_HOT != EXTREMELY_HIGH...
          | && state_ECT != HIGH...
          | && state_ECT != EXTREMELY_HIGH]
      Control.AutoShift_TrackTensioning.Active -> Control.AutoShift_TrackTensioning.Inactive : [state_Console != ACTIVE...
          | || state_HOT == HIGH...
          | || state_HOT == EXTREMELY_HIGH...
          | || state_ECT == HIGH...
          | || state_ECT == EXTREMELY_HIGH]
    state AutoShift
      state Inactive
        en:
        cmd_TwoSpeed = INACTIVE;
        state_TwoSpeedIcon = INACTIVE;
      state Active
        state Autoshift_Inactive
          en:
          cmd_TwoSpeed = LOW;
          state_TwoSpeedIcon = INACTIVE;
        state Autoshift_Active
          en:
          state_TwoSpeedIcon = ACTIVE;
          state Low_No_Travel
            en:
            cmd_TwoSpeed = LOW;
          state Delay
          state High
            en:
            cmd_TwoSpeed = HIGH;
          state Low_Travel
            en:
            cmd_TwoSpeed = LOW;
          (default) -> Low_No_Travel
          Delay -> Low_No_Travel [order 2] : [din_TravelPressureSwitch == INACTIVE]
          Delay -> Control.AutoShift.Active.Autoshift_Active.High [order 1] : [after(CD_AUTOSHIFT_TRAVEL_DELAY, tick)]
          (junction 2705) -> Delay [order 1] : [CD_AUTOSHIFT_TRAVEL_DELAY > 0]
          Low_No_Travel -> (junction 2705) : [din_TravelPressureSwitch == ACTIVE]
          (junction 2705) -> (junction 2706) [order 2]
          (junction 2706) -> Control.AutoShift.Active.Autoshift_Active.High
          Control.AutoShift.Active.Autoshift_Active.High -> Low_No_Travel [order 2] : [din_TravelPressureSwitch == INACTIVE]
          Control.AutoShift.Active.Autoshift_Active.Low_Travel -> Low_No_Travel [order 1] : [din_TravelPressureSwitch == INACTIVE]
          Control.AutoShift.Active.Autoshift_Active.High -> Control.AutoShift.Active.Autoshift_Active.Low_Travel [order 1] : [pressure_LoadSense > P_high...
              | && state_LoadSense == NORMAL]
          Control.AutoShift.Active.Autoshift_Active.Low_Travel -> Control.AutoShift.Active.Autoshift_Active.High [order 2] : [pressure_LoadSense < P_low...
              | || state_LoadSense != NORMAL]
        (default) -> Autoshift_Inactive
        Autoshift_Inactive -> (junction 2692) : [risingedge_TwoSpeed]
        (junction 2694) -> Autoshift_Inactive [order 2]
        (junction 2695) -> Autoshift_Inactive : {
            | state_Buzzer = SINGLE_SHORT_BEEP;
            | }
        (junction 2692) -> (junction 2693) [order 1] : [CD_TWOSPEED_BEEP_FUNCTION != NON_FUNCTIONAL]
        (junction 2694) -> (junction 2695) [order 1] : [CD_TWOSPEED_BEEP_FUNCTION != NON_FUNCTIONAL]
        (junction 2692) -> Autoshift_Active [order 2]
        (junction 2693) -> Autoshift_Active : {
            | state_Buzzer = DOUBLE_SHORT_BEEP;
            | }
        Autoshift_Active -> (junction 2694) : [risingedge_TwoSpeed]
      (default) -> Control.AutoShift.Inactive
      Control.AutoShift.Inactive -> Control.AutoShift.Active : [state_Console == ACTIVE...
          | && state_HOT != HIGH...
          | && state_HOT != EXTREMELY_HIGH...
          | && state_ECT != HIGH...
          | && state_ECT != EXTREMELY_HIGH]
      Control.AutoShift.Active -> Control.AutoShift.Inactive : [state_Console != ACTIVE...
          | || state_HOT == HIGH...
          | || state_HOT == EXTREMELY_HIGH...
          | || state_ECT == HIGH...
          | || state_ECT == EXTREMELY_HIGH]
    state TrackTensioning
      state Inactive
        en:
        cmd_TwoSpeed = INACTIVE;
        state_TwoSpeedIcon = INACTIVE;
      state Active
        state Low
          en:
          state_TwoSpeedIcon = INACTIVE;
          state Normal
            en:
            cmd_TwoSpeed = LOW;
          state Track_Tensioning
            en:
            cmd_TwoSpeed = HIGH;
          (default) -> Control.TrackTensioning.Active.Low.Normal
          Control.TrackTensioning.Active.Low.Normal -> Control.TrackTensioning.Active.Low.Track_Tensioning : [din_TravelPressureSwitch == INACTIVE]
          Control.TrackTensioning.Active.Low.Track_Tensioning -> Control.TrackTensioning.Active.Low.Normal : [din_TravelPressureSwitch == ACTIVE]
        state High
          en:
          cmd_TwoSpeed = HIGH;
          state_TwoSpeedIcon = ACTIVE;
        (default) -> Control.TrackTensioning.Active.Low
        Control.TrackTensioning.Active.Low -> (junction 2721) : [risingedge_TwoSpeed]
        (junction 2723) -> Control.TrackTensioning.Active.Low [order 2]
        (junction 2724) -> Control.TrackTensioning.Active.Low : {
            | state_Buzzer = SINGLE_SHORT_BEEP;
            | }
        (junction 2721) -> (junction 2722) [order 1] : [CD_TWOSPEED_BEEP_FUNCTION != NON_FUNCTIONAL]
        (junction 2723) -> (junction 2724) [order 1] : [CD_TWOSPEED_BEEP_FUNCTION != NON_FUNCTIONAL]
        (junction 2721) -> Control.TrackTensioning.Active.High [order 2]
        (junction 2722) -> Control.TrackTensioning.Active.High : {
            | state_Buzzer = DOUBLE_SHORT_BEEP;
            | }
        Control.TrackTensioning.Active.High -> (junction 2723) : [risingedge_TwoSpeed]
      (default) -> Control.TrackTensioning.Inactive
      Control.TrackTensioning.Active -> Control.TrackTensioning.Inactive : [state_Console != ACTIVE]
      Control.TrackTensioning.Inactive -> Control.TrackTensioning.Active : [state_Console == ACTIVE]
    state Normal
      state Inactive
        en:
        cmd_TwoSpeed = INACTIVE;
        state_TwoSpeedIcon = INACTIVE;
      state Active
        state Low
          en:
          cmd_TwoSpeed = LOW;
          state_TwoSpeedIcon = INACTIVE;
        state High
          en:
          cmd_TwoSpeed = HIGH;
          state_TwoSpeedIcon = ACTIVE;
        (default) -> Control.Normal.Active.Low
        Control.Normal.Active.Low -> (junction 2740) : [risingedge_TwoSpeed]
        (junction 2742) -> Control.Normal.Active.Low [order 2]
        (junction 2743) -> Control.Normal.Active.Low : {
            | state_Buzzer = SINGLE_SHORT_BEEP;
            | }
        (junction 2740) -> (junction 2741) [order 1] : [CD_TWOSPEED_BEEP_FUNCTION != NON_FUNCTIONAL]
        (junction 2742) -> (junction 2743) [order 1] : [CD_TWOSPEED_BEEP_FUNCTION != NON_FUNCTIONAL]
        (junction 2740) -> Control.Normal.Active.High [order 2]
        (junction 2741) -> Control.Normal.Active.High : {
            | state_Buzzer = DOUBLE_SHORT_BEEP;
            | }
        Control.Normal.Active.High -> (junction 2742) : [risingedge_TwoSpeed]
      (default) -> Control.Normal.Inactive
      Control.Normal.Active -> Control.Normal.Inactive : [state_Console != ACTIVE...
          | || state_HOT == HIGH...
          | || state_HOT == EXTREMELY_HIGH...
          | || state_ECT == HIGH...
          | || state_ECT == EXTREMELY_HIGH]
      Control.Normal.Inactive -> Control.Normal.Active : [state_Console == ACTIVE...
          | && state_HOT != HIGH...
          | && state_HOT != EXTREMELY_HIGH...
          | && state_ECT != HIGH...
          | && state_ECT != EXTREMELY_HIGH]
    (default) -> NonFunctional
    NonFunctional -> (junction 2652)
    (junction 2652) -> AutoShift_TrackTensioning [order 1] : [CD_AUTOSHIFT_FUNCTION != NON_FUNCTIONAL...
        | && CD_TRACKTENSION_FUNCTION != NON_FUNCTIONAL]
    (junction 2652) -> (junction 2653) [order 2]
    (junction 2653) -> AutoShift [order 1] : [CD_AUTOSHIFT_FUNCTION != NON_FUNCTIONAL]
    (junction 2653) -> (junction 2654) [order 2]
    (junction 2654) -> TrackTensioning [order 1] : [CD_TRACKTENSION_FUNCTION != NON_FUNCTIONAL]
    (junction 2654) -> Control.Normal [order 2]
  state Two_Speed_Command [parallel]
    settings: executionOrder=2
    state Low
      en:
      duty_TwoSpeed = INACTIVE;
    state High
      en:
      duty_TwoSpeed = FULL_ON;
    (default) -> Two_Speed_Command.Low
    Two_Speed_Command.High -> Two_Speed_Command.Low : [cmd_TwoSpeed != HIGH...
        | || state_Engine == ENGINE_CRANKING...
        | || state_HydEnable != ACTIVE]
    Two_Speed_Command.Low -> Two_Speed_Command.High : [cmd_TwoSpeed == HIGH...
        | && state_Engine != ENGINE_CRANKING...
        | && state_HydEnable == ACTIVE]
  data: risingedge_TwoSpeed input uint8; cmd_TwoSpeed local uint8; FULL_ON parameter fixdt(0,16,16); EXTREMELY_HIGH parameter uint8; state_ECT input uint8; HIGH parameter uint8; INACTIVE parameter uint8; LOW parameter uint8; state_Console input uint8; state_Engine input uint8; ENGINE_CRANKING parameter uint8; state_HydEnable input uint8; NORMAL parameter uint8; CD_AUTOSHIFT_FUNCTION parameter uint8; pressure_LoadSense input uint16; din_TravelPressureSwitch input boolean; state_TwoSpeedIcon output uint8; duty_TwoSpeed output fixdt(0,16,16); CD_AUTOSHIFT_TRAVEL_DELAY parameter uint8; NON_FUNCTIONAL parameter uint8; state_LoadSense input uint8; state_HOT input uint8; P_high input uint16; P_low input uint16; ACTIVE parameter uint8; CD_TWOSPEED_BEEP_FUNCTION parameter uint8; state_Buzzer data_store_memory double; DOUBLE_SHORT_BEEP parameter uint8; SINGLE_SHORT_BEEP parameter uint8; CD_TRACKTENSION_FUNCTION parameter uint8

== stateflow chart MX_Gtwy_Control/Inputs/EMEA_Right_Control/Right Pot/Right_Pot ==
  state Right_Pot_Neutral_Check [parallel]
    settings: executionOrder=1
    state Initialize
      en:
      state_RightPotCal = NORMAL;
      offset_RightPotCal = 0;
    state WaitForPress
    state Wait_For_New_Cal
    note: if we are already NOT_IN_NEUTRAL
        | be sure to not beep again
    note: set the triple beep here instead of in the fault code
        | to be sure other aux beeps don't override the fault code beep
    note: Note - we dont want to check for neutral fault with aux button press if offset is on right,
        | but we do want to be able to recover with aux button press
    (default) -> Initialize
    Initialize -> WaitForPress
    WaitForPress -> (junction 2807) [order 1] : [(aux_Flow == AUX_FLOW_LOW || aux_Flow == AUX_FLOW_MEDIUM || aux_Flow == AUX_FLOW_STANDARD || aux_Flow == AUX_FLOW_HIGH)...
        | && state_Console == ACTIVE...
        | && state_Engine != ENGINE_CRANKING...
        | && state_RightPot != OORL...
        | && state_RightPot != OORH...
        | && state_SensorSupply == NORMAL]
    (junction 2807) -> (junction 2808)
    (junction 2808) -> (junction 2809) [order 1] : [rm_RightPot >= CD_EMEA_POT_CAL_DEADBAND_LOW...
        |  && rm_RightPot <= CD_EMEA_POT_CAL_DEADBAND_HIGH]
    (junction 2809) -> (junction 2813) : {
        | state_RightPotCal = NORMAL;
        | offset_RightPotCal = int16(CD_AUXPOT_CENTER_RATIO) - rm_RightPot;
        | }
    (junction 2812) -> (junction 2807)
    (junction 2808) -> (junction 2810) [order 2]
    (junction 2810) -> (junction 2811) [order 1] : [state_RightPot != NOT_IN_NEUTRAL]
    (junction 2810) -> (junction 2814) [order 2] : {
        | state_Buzzer = BEEP_NONE;
        | }
    (junction 2811) -> (junction 2815) : {
        | state_RightPotCal = NOT_IN_NEUTRAL;
        | offset_RightPotCal = 0;
        | state_Buzzer = TRIPLE_SHORT_BEEP;
        | }
    WaitForPress -> (junction 2812) [order 2] : [(aux_DebounceButton || aux_DetentHold)...
        | && !aux_Inhibit...
        | && mode_RightControl != MODE_RIGHT_OFFSET...
        | && (state_AuxEnable == INACTIVE || aux_Flow == AUX_FLOW_INACTIVE)...
        | && state_RightPot != OORL...
        | && state_RightPot != OORH...
        | && state_SensorSupply == NORMAL]
    (junction 2816) -> (junction 2812)
    (junction 2813) -> Wait_For_New_Cal
    (junction 2814) -> (junction 2815)
    (junction 2815) -> Wait_For_New_Cal
    Wait_For_New_Cal -> (junction 2816) [order 2] : [(aux_DebounceButton || aux_DetentHold)...
        | && !aux_Inhibit...
        | && (mode_RightControl != MODE_RIGHT_OFFSET || state_RightPotCal == NOT_IN_NEUTRAL)...
        | && (state_AuxEnable == INACTIVE || aux_Flow == AUX_FLOW_INACTIVE)...
        | && state_RightPot != OORL...
        | && state_RightPot != OORH...
        | && state_SensorSupply == NORMAL]
    (junction 2817) -> (junction 2816)
    WaitForPress -> (junction 2817) [order 3] : [check_RightNeutral...
        | && state_RightPot != OORL...
        | && state_RightPot != OORH...
        | && state_SensorSupply == NORMAL]
    Wait_For_New_Cal -> (junction 2817) [order 3] : [check_RightNeutral]
    Wait_For_New_Cal -> WaitForPress [order 1] : [state_Console != ACTIVE...
        | || state_Engine == ENGINE_CRANKING...
        | || state_RightPot == OORL ...
        | || state_RightPot ==OORH ...
        | || state_SensorSupply != NORMAL]
  state Right_Pot_State [parallel]
    settings: executionOrder=2
    state Supply_OK
      state NORMAL
        en:
        position_RightPotIn = rm_RightPot + offset_RightPotCal;
        position_RightPotOut = position_RightPotIn;
        du:
        position_RightPotIn = rm_RightPot + offset_RightPotCal;
        position_RightPotOut = position_RightPotIn;
        (history)
        state Neutral
          en:
          state_RightPot = NEUTRAL;
        state Rod_Active
          en:
          state_RightPot =...
          ROD_ACTIVE;
        state Base_Active
          en:
          state_RightPot =...
          BASE_ACTIVE;
        (default) -> Neutral
        Neutral -> Base_Active [order 2] : [position_RightPotIn < base_Threshold]
        Neutral -> Rod_Active [order 1] : [position_RightPotIn > rod_Threshold]
        Rod_Active -> Neutral [order 2] : [position_RightPotIn <= rod_Threshold]
        Base_Active -> Neutral [order 2] : [position_RightPotIn >= base_Threshold]
        Rod_Active -> Base_Active [order 1] : [position_RightPotIn <= rod_Threshold...
            |  && position_RightPotIn < base_Threshold]
        Base_Active -> Rod_Active [order 1] : [position_RightPotIn >= rod_Threshold...
            |  && position_RightPotIn > base_Threshold]
      state OORH
        en:
        state_RightPot = OORH;
      state debounce_OORH
        en:
        position_RightPotOut =...
        CD_AUXPOT_CENTER_RATIO;
      state OORL
        en:
        state_RightPot = OORL;
      state debounce_OORL
        en:
        position_RightPotOut =...
        CD_AUXPOT_CENTER_RATIO;
      state NOT_IN_NEUTRAL
        en:
        state_RightPot = NOT_IN_NEUTRAL;
        position_RightPotOut =...
        CD_AUXPOT_CENTER_RATIO;
      (default) -> NORMAL
      NORMAL -> debounce_OORH [order 1] : [raw_RightPot > CD_AUXPOT_OORH]
      debounce_OORH -> OORH [order 1] : [after(CD_AUXPOT_OOR_DB,tick)]
      debounce_OORH -> NORMAL [order 2] : [raw_RightPot <= CD_AUXPOT_OORH]
      NORMAL -> debounce_OORL [order 2] : [raw_RightPot < CD_AUXPOT_OORL]
      debounce_OORL -> OORL [order 1] : [after(CD_AUXPOT_OOR_DB,tick)]
      debounce_OORL -> NORMAL [order 2] : [raw_RightPot >= CD_AUXPOT_OORL]
      NORMAL -> NOT_IN_NEUTRAL [order 3] : [state_RightPotCal == NOT_IN_NEUTRAL]
      NOT_IN_NEUTRAL -> NORMAL : [state_RightPotCal != NOT_IN_NEUTRAL]
    state Supply_Fault
      en:
      state_RightPot = NORMAL;
      position_RightPotOut =...
      CD_AUXPOT_CENTER_RATIO;
    (default) -> Supply_OK : {
        | base_Threshold = CD_AUXPOT_CENTER_RATIO - CD_AUXPOT_DEADBAND;
        | rod_Threshold = CD_AUXPOT_CENTER_RATIO + CD_AUXPOT_DEADBAND;
        | }
    Supply_OK -> Supply_Fault : [state_SensorSupply != NORMAL]
    Supply_Fault -> Supply_OK : [state_SensorSupply == NORMAL]
  data: aux_DebounceButton input uint8; aux_Flow input uint8; state_AuxEnable input uint8; aux_DetentHold input uint8; state_RightPot output uint8; position_RightPotOut output int16; position_RightPotIn local int16 size=1; offset_RightPotCal local int16; state_RightPotCal local uint8; CD_AUXPOT_CENTER_RATIO parameter uint16; CD_EMEA_POT_CAL_DEADBAND_HIGH parameter uint16; CD_EMEA_POT_CAL_DEADBAND_LOW parameter uint16; CD_AUXPOT_OORH parameter uint16; CD_AUXPOT_OORL parameter uint16; NOT_IN_NEUTRAL parameter uint8; OORH parameter uint8; OORL parameter uint8; CD_AUXPOT_OOR_DB parameter uint8; BASE_ACTIVE parameter uint8; base_Threshold local uint16; CD_AUXPOT_DEADBAND parameter uint8; NEUTRAL parameter uint8; ROD_ACTIVE parameter uint8; rod_Threshold local uint16; NORMAL parameter uint8; INACTIVE parameter uint8; ACTIVE parameter uint8; state_Console input uint8; state_Engine input uint8; state_SensorSupply input uint8; aux_Inhibit input boolean; check_RightNeutral input boolean; mode_RightControl input uint8; raw_RightPot input uint16; rm_RightPot input int16; ENGINE_CRANKING parameter uint8; TRIPLE_SHORT_BEEP parameter uint8; state_Buzzer data_store_memory double; BEEP_NONE parameter uint8; AUX_FLOW_LOW parameter uint8; AUX_FLOW_MEDIUM parameter uint8; AUX_FLOW_STANDARD parameter uint8; AUX_FLOW_HIGH parameter uint8; AUX_FLOW_INACTIVE parameter uint8; MODE_RIGHT_OFFSET parameter uint8
